simulink library "TripedLibraryMAX"  (R2021a)
settings: Solver=VariableStepAuto  StartTime=0.0  StopTime=10.0  RelTol=1e-3
undefined symbols (not defined in the file or in its folder): X, Y, Z
libraries: sm_lib x100, nesl_utility x31, $bdroot x27, robotlib x27, simulink x6
(part 3 of 6)

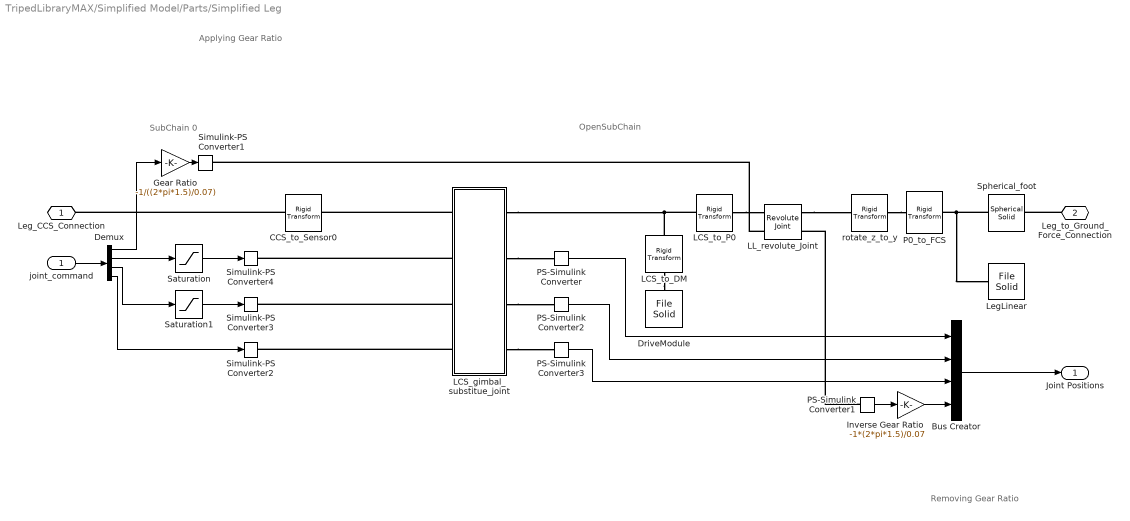
== system TripedLibraryMAX/Simplified Model/Parts/Simplified Leg ==
  note: Removing Gear Ratio
  note: Applying Gear Ratio
  note: SubChain 0
  note: OpenSubChain
  joint_command : Inport
  Bus Creator : BusCreator  Inputs=4
  CCS_to_Sensor0 : sm_lib/Frames and Transforms/Rigid Transform  TranslationMethod=Cartesian  TranslationStandardOffset=0  TranslationLengthUnit=mm  TranslationStandardAxis=+Z  TranslationCartesianOffset=[70 0 53]+[195 0 -39]  TranslationROffset=0  TranslationROffsetUnits=m  TranslationZOffset=0  TranslationZOffsetUnits=m  TranslationThetaOffset=0  TranslationThetaOffsetUnits=deg  RotationMethod=None  RotationAngleUnits=deg  RotationStandardAxis=+Z  RotationAngle=0.0  RotationArbitraryAxis=[0 0 1]  FollAlignAxisA=+X  BaseAlignAxisA=+Y  FollAlignAxisB=+Y  BaseAlignAxisB=+Z  RotationSequenceAxes=FollowerAxes  RotationSequence=XYX  RotationSequenceAngles=[0 0 0]  RotationSequenceAnglesUnits=deg  RotationMatrix=[1 0 0; 0 1 0; 0 0 1]
  Demux : Demux
  DriveModule : sm_lib/Body Elements/File Solid  ExtGeomFileName=./triped-files/TriPed_Bein_Antriebsmodul.stl  UnitType=Custom  ExtGeomFileUnits=mm  StepReaderType=HEX  ExportConvexHullGeometry=off  InertiaType=Custom  Mass=1.908  MassUnits=kg  CenterOfMass=[25.9 50.7 45.5]  CenterOfMassUnits=mm  MomentsOfInertia=[0.005 0.007 0.008]  MomentsOfInertiaUnits=kg*m^2  ProductsOfInertia=[0 0 0]  ProductsOfInertiaUnits=kg*m^2  BasedOnType=DensityFromFile  Density=1000  DensityUnits=kg/m^3  DoExposeReferenceFrame=on  SerializedFrames=<Frames/>  GraphicType=FromGeometry  MarkerShape=Sphere  MarkerSize=10  GraphicVisPropType=SimpleVisualProperties  GraphicDiffuseColor=[0.0 0.39607844 0.44705883]  GraphicSpecularColor=[0.5 0.5 0.5 1.0]  GraphicAmbientColor=[0.15 0.15 0.15 1.0]  GraphicEmissiveColor=[0.0 0.0 0.0 1.0]  GraphicShininess=75  GraphicOpacity=0.75
  Gear Ratio : Gain  Gain=-1/((2*pi*1.5)/0.07)
  Inverse Gear Ratio : Gain  Gain=-1*(2*pi*1.5)/0.07
  LCS_gimbal_substitue_joint : Subsystem
  LCS_to_DM : sm_lib/Frames and Transforms/Rigid Transform  TranslationMethod=Cartesian  TranslationStandardOffset=0  TranslationLengthUnit=mm  TranslationStandardAxis=+Z  TranslationCartesianOffset=[25.9 145 37]  TranslationROffset=0  TranslationROffsetUnits=m  TranslationZOffset=0  TranslationZOffsetUnits=m  TranslationThetaOffset=0  TranslationThetaOffsetUnits=deg  RotationMethod=StandardAxis  RotationAngleUnits=deg  RotationStandardAxis=+Z  RotationAngle=-90  RotationArbitraryAxis=[0 0 1]  FollAlignAxisA=+X  BaseAlignAxisA=+Y  FollAlignAxisB=+Y  BaseAlignAxisB=+Z  RotationSequenceAxes=FollowerAxes  RotationSequence=XYX  RotationSequenceAngles=[0 0 0]  RotationSequenceAnglesUnits=deg  RotationMatrix=[1 0 0; 0 1 0; 0 0 1]
  LCS_to_P0 : sm_lib/Frames and Transforms/Rigid Transform  TranslationMethod=Cartesian  TranslationStandardOffset=0  TranslationLengthUnit=mm  TranslationStandardAxis=+Z  TranslationCartesianOffset=[1640 0 -37]  TranslationROffset=0  TranslationROffsetUnits=m  TranslationZOffset=0  TranslationZOffsetUnits=m  TranslationThetaOffset=0  TranslationThetaOffsetUnits=deg  RotationMethod=StandardAxis  RotationAngleUnits=deg  RotationStandardAxis=+X  RotationAngle=-90  RotationArbitraryAxis=[0 0 1]  FollAlignAxisA=+X  BaseAlignAxisA=+Y  FollAlignAxisB=+Y  BaseAlignAxisB=+Z  RotationSequenceAxes=FollowerAxes  RotationSequence=XYX  RotationSequenceAngles=[0 0 0]  RotationSequenceAnglesUnits=deg  RotationMatrix=[1 0 0; 0 1 0; 0 0 1]
  LL_revolute_Joint : sm_lib/Joints/Revolute Joint  JointMode=Normal  SenseConstraintForce=off  SenseConstraintForceX=off  SenseConstraintForceY=off  SenseConstraintForceZ=off  SenseConstraintTorque=off  SenseConstraintTorqueX=off  SenseConstraintTorqueY=off  SenseConstraintTorqueZ=off  SenseTotalForce=off  SenseTotalForceX=off  SenseTotalForceY=off  SenseTotalForceZ=off  SenseTotalTorque=off  SenseTotalTorqueX=off  SenseTotalTorqueY=off  SenseTotalTorqueZ=off  CompositeWrenchDir=FollowerOnBase  CompositeWrenchFrame=BaseFrame  EquilibriumPosition=0  EquilibriumPositionUnits=deg  SpringStiffness=0  SpringStiffnessUnits=N*m/deg  DampingCoefficient=0  DampingCoefficientUnits=N*m/(deg/s)  TorqueActuationMode=ComputedTorque  MotionActuationMode=InputMotion  SensePosition=on  SenseVelocity=off  SenseAcceleration=off  SenseTorqueForce=off  SenseLowerLimitTorqueForce=off  SenseUpperLimitTorqueForce=off  LowerLimitSpecify=off  LowerLimitBound=-60  LowerLimitBoundUnits=deg  LowerLimitStiffness=1e4  LowerLimitStiffnessUnits=N*m/deg  LowerLimitDamping=10  LowerLimitDampingUnits=N*m/(deg/s)  LowerLimitTransitionRegionWidth=0.1  LowerLimitTransitionRegionWidthUnits=deg  UpperLimitSpecify=off  UpperLimitBound=30  UpperLimitBoundUnits=deg  UpperLimitStiffness=1e4  UpperLimitStiffnessUnits=N*m/deg  UpperLimitDamping=1e4  UpperLimitDampingUnits=N*m/(deg/s)  UpperLimitTransitionRegionWidth=0.1  UpperLimitTransitionRegionWidthUnits=deg  PositionTargetSpecify=off  PositionTargetPriority=High  PositionTargetValue=0  PositionTargetValueUnits=deg  VelocityTargetSpecify=off  VelocityTargetPriority=High  VelocityTargetValue=0  VelocityTargetValueUnits=deg/s
  LegLinear : sm_lib/Body Elements/File Solid  ExtGeomFileName=./triped-files/TriPed_Bein_Linearteil_überarbeitet.stl  UnitType=Custom  ExtGeomFileUnits=mm  StepReaderType=HEX  ExportConvexHullGeometry=on  InertiaType=Custom  Mass=0.503  MassUnits=kg  CenterOfMass=[94.275 0 452.725]  CenterOfMassUnits=mm  MomentsOfInertia=[0.0006157 0.047 0.047]  MomentsOfInertiaUnits=kg*m^2  ProductsOfInertia=[0 0 0]  ProductsOfInertiaUnits=kg*m^2  BasedOnType=DensityFromFile  Density=1000  DensityUnits=kg/m^3  DoExposeReferenceFrame=on  SerializedFrames=<Frames/>  GraphicType=FromGeometry  MarkerShape=Sphere  MarkerSize=10  GraphicVisPropType=SimpleVisualProperties  GraphicDiffuseColor=[0.60784316 0.6509804 0.05882353]  GraphicSpecularColor=[0.5 0.5 0.5 1.0]  GraphicAmbientColor=[0.15 0.15 0.15 1.0]  GraphicEmissiveColor=[0.0 0.0 0.0 1.0]  GraphicShininess=75  GraphicOpacity=1.0
  P0_to_FCS : sm_lib/Frames and Transforms/Rigid Transform  TranslationMethod=StandardAxis  TranslationStandardOffset=-1500  TranslationLengthUnit=mm  TranslationStandardAxis=+X  TranslationCartesianOffset=[0 0 0]  TranslationROffset=0  TranslationROffsetUnits=m  TranslationZOffset=0  TranslationZOffsetUnits=m  TranslationThetaOffset=0  TranslationThetaOffsetUnits=deg  RotationMethod=None  RotationAngleUnits=deg  RotationStandardAxis=+Z  RotationAngle=0.0  RotationArbitraryAxis=[0 0 1]  FollAlignAxisA=+X  BaseAlignAxisA=+Y  FollAlignAxisB=+Y  BaseAlignAxisB=+Z  RotationSequenceAxes=FollowerAxes  RotationSequence=XYX  RotationSequenceAngles=[0 0 0]  RotationSequenceAnglesUnits=deg  RotationMatrix=[1 0 0; 0 1 0; 0 0 1]
  PS-Simulink Converter{,1..3} (4 alike) : nesl_utility/PS-Simulink Converter  PhysicalDomain=network_engine_domain  SubClassName=ps_output  LeftPortType=input  RightPortType=output  PseudoPeriodic=off  Frequencies=[]  Unit=inherit  AffineConversion=off
      settings: SourceProductBaseCode=PW,SS
  Saturation : Saturate  UpperLimit=0.523599  LowerLimit=-0.523599
  Saturation1 : Saturate  UpperLimit=0.523599  LowerLimit=-0.523599
  Simulink-PS Converter{1..4} (4 alike) : nesl_utility/Simulink-PS Converter  PhysicalDomain=network_engine_domain  SubClassName=ps_input  LeftPortType=input  RightPortType=output  PseudoPeriodic=off  Frequencies=[]  NoiseDistribution=none  NoiseParameters=[]  Unit=1  AffineConversion=off  FilteringAndDerivatives=filter  SimscapeFilterOrder=2  InputFilterTimeConstant=0.01  UdotUserProvided=0
      settings: SourceProductBaseCode=PW,SS
  Spherical_foot : sm_lib/Body Elements/Spherical Solid  GraphicAmbientColor=[0.15 0.15 0.15 1.0]  BasedOnType=Density  CenterOfMass=[0 0 0]  CenterOfMassUnits=m  GraphicDiffuseColor=[1.0 0.63529414 0.0]  Density=0  DensityUnits=kg/m^3  DoExposeReferenceFrame=on  GraphicEmissiveColor=[0.0 0.0 0.0 1.0]  ExportEntireGeometry=on  SerializedExposedGeometries=<ExposedGeometries><ExposedGeometry><RawGeometryId>entire</RawGeometryId><ExposedGeometryId>G</ExposedGeometryId></ExposedGeometry></ExposedGeometries>  SerializedFrames=<Frames/>  MarkerShape=Sphere  MarkerSize=10  GraphicType=FromGeometry  InertiaType=CalculateFromGeometry  Mass=1  MassUnits=kg  MomentsOfInertia=[1 1 1]  MomentsOfInertiaUnits=kg*m^2  GraphicOpacity=1.0  ProductsOfInertia=[0 0 0]  ProductsOfInertiaUnits=kg*m^2  GraphicShininess=75  GraphicSpecularColor=[0.5 0.5 0.5 1.0]  SphereRadius=0.03  SphereRadiusUnits=m  GraphicVisPropType=SimpleVisualProperties
  rotate_z_to_y : sm_lib/Frames and Transforms/Rigid Transform  TranslationMethod=None  TranslationStandardOffset=0  TranslationLengthUnit=m  TranslationStandardAxis=+Z  TranslationCartesianOffset=[0 0 0]  TranslationROffset=0  TranslationROffsetUnits=m  TranslationZOffset=0  TranslationZOffsetUnits=m  TranslationThetaOffset=0  TranslationThetaOffsetUnits=deg  RotationMethod=StandardAxis  RotationAngleUnits=deg  RotationStandardAxis=+X  RotationAngle=90  RotationArbitraryAxis=[0 0 1]  FollAlignAxisA=+X  BaseAlignAxisA=+Y  FollAlignAxisB=+Y  BaseAlignAxisB=+Z  RotationSequenceAxes=FollowerAxes  RotationSequence=XYX  RotationSequenceAngles=[0 0 0]  RotationSequenceAnglesUnits=deg  RotationMatrix=[1 0 0; 0 1 0; 0 0 1]
  Leg_CCS_Connection : PMIOPort  Side=Left
  Leg_to_Ground_Force_Connection : PMIOPort  Port=2  Side=Left
  Joint Positions : Outport
  wires:
  PS-Simulink Converter3/1 -> Bus Creator/3
  PS-Simulink Converter2/1 -> Bus Creator/2
  Inverse Gear Ratio/1 -> Bus Creator/4
  Demux/4 -> Simulink-PS Converter2/1
  Demux/3 -> Saturation1/1
  PS-Simulink Converter/1 -> Bus Creator/1
  Gear Ratio/1 -> Simulink-PS Converter1/1
  Bus Creator/1 -> Joint Positions/1
  PS-Simulink Converter1/1 -> Inverse Gear Ratio/1
  joint_command/1 -> Demux/1
  Demux/1 -> Gear Ratio/1
  Demux/2 -> Saturation/1
  Saturation/1 -> Simulink-PS Converter4/1
  Saturation1/1 -> Simulink-PS Converter3/1
  connections:
  P0_to_FCS/RConn1 -- Spherical_foot/RConn1 -- LegLinear/RConn1
  LCS_to_P0/LConn1 -- LCS_gimbal_substitue_joint/RConn1 -- LCS_to_DM/RConn1
  rotate_z_to_y/RConn1 -- P0_to_FCS/LConn1
  LCS_gimbal_substitue_joint/LConn4 -- Simulink-PS Converter2/RConn1
  LCS_gimbal_substitue_joint/RConn3 -- PS-Simulink Converter2/LConn1
  LCS_gimbal_substitue_joint/LConn2 -- Simulink-PS Converter4/RConn1
  LL_revolute_Joint/LConn2 -- Simulink-PS Converter1/RConn1
  LCS_gimbal_substitue_joint/LConn3 -- Simulink-PS Converter3/RConn1
  LCS_to_P0/RConn1 -- LL_revolute_Joint/LConn1
  LCS_gimbal_substitue_joint/RConn2 -- PS-Simulink Converter/LConn1
  LCS_gimbal_substitue_joint/RConn4 -- PS-Simulink Converter3/LConn1
  LCS_to_DM/LConn1 -- DriveModule/RConn1
  Leg_to_Ground_Force_Connection/RConn1 -- Spherical_foot/LConn1
  LL_revolute_Joint/RConn1 -- rotate_z_to_y/LConn1
  LCS_gimbal_substitue_joint/LConn1 -- CCS_to_Sensor0/RConn1
  PS-Simulink Converter1/LConn1 -- LL_revolute_Joint/RConn2
  CCS_to_Sensor0/LConn1 -- Leg_CCS_Connection/RConn1

== system TripedLibraryMAX/Simplified Model/Simplified TriPed/Chassis ==
  Chassis : sm_lib/Body Elements/File Solid  ExtGeomFileName=./triped-files/TriPed_Chassis_überarbeitet.stl  UnitType=Custom  ExtGeomFileUnits=mm  StepReaderType=HEX  ExportConvexHullGeometry=off  InertiaType=Custom  Mass=10.414  MassUnits=kg  CenterOfMass=[0 0 10.44]  CenterOfMassUnits=mm  MomentsOfInertia=[0.127 0.127 0.209]  MomentsOfInertiaUnits=kg*m^2  ProductsOfInertia=[0 0 0]  ProductsOfInertiaUnits=kg*m^2  BasedOnType=DensityFromFile  Density=1000  DensityUnits=kg/m^3  DoExposeReferenceFrame=on  SerializedFrames=<Frames/>  GraphicType=FromGeometry  MarkerShape=Sphere  MarkerSize=10  GraphicVisPropType=SimpleVisualProperties  GraphicDiffuseColor=[1.0 0.63529414 0.0]  GraphicSpecularColor=[0.5 0.5 0.5 1.0]  GraphicAmbientColor=[0.15 0.15 0.15 1.0]  GraphicEmissiveColor=[0.0 0.0 0.0 1.0]  GraphicShininess=75  GraphicOpacity=1
  visualize CCS : Subsystem
  " Chassis_connection" : PMIOPort  Side=Left
  Forward Kin Visual : PMIOPort  Port=2  Side=Right
  connections:
  Chassis/RConn1 -- visualize CCS/LConn1 -- Forward Kin Visual/RConn1 -- " Chassis_connection"/RConn1

== system TripedLibraryMAX/Simplified Model/Simplified TriPed (Kinematics)/Chassis ==
  Chassis : sm_lib/Body Elements/File Solid  ExtGeomFileName=./triped-files/TriPed_Chassis_überarbeitet.stl  UnitType=Custom  ExtGeomFileUnits=mm  StepReaderType=HEX  ExportConvexHullGeometry=off  InertiaType=Custom  Mass=10.414  MassUnits=kg  CenterOfMass=[0 0 10.44]  CenterOfMassUnits=mm  MomentsOfInertia=[0.127 0.127 0.209]  MomentsOfInertiaUnits=kg*m^2  ProductsOfInertia=[0 0 0]  ProductsOfInertiaUnits=kg*m^2  BasedOnType=DensityFromFile  Density=1000  DensityUnits=kg/m^3  DoExposeReferenceFrame=on  SerializedFrames=<Frames/>  GraphicType=FromGeometry  MarkerShape=Sphere  MarkerSize=10  GraphicVisPropType=SimpleVisualProperties  GraphicDiffuseColor=[1.0 0.63529414 0.0]  GraphicSpecularColor=[0.5 0.5 0.5 1.0]  GraphicAmbientColor=[0.15 0.15 0.15 1.0]  GraphicEmissiveColor=[0.0 0.0 0.0 1.0]  GraphicShininess=75  GraphicOpacity=1
  visualize CCS : Subsystem
  " Chassis_connection" : PMIOPort  Side=Left
  Forward Kin Visual : PMIOPort  Port=2  Side=Right
  connections:
  Chassis/RConn1 -- visualize CCS/LConn1 -- Forward Kin Visual/RConn1 -- " Chassis_connection"/RConn1

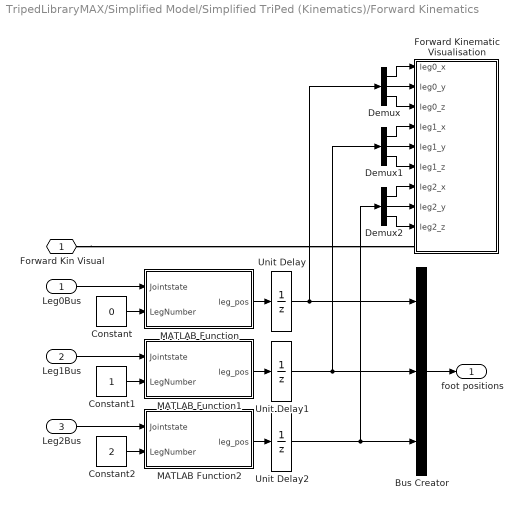
== system TripedLibraryMAX/Simplified Model/Simplified TriPed (Kinematics)/Forward Kinematics ==
  Leg0Bus : Inport
  Leg1Bus : Inport  Port=2
  Leg2Bus : Inport  Port=3
  Bus Creator : BusCreator  Inputs=3
  Constant : Constant  Value=0
  Constant1 : Constant
  Constant2 : Constant  Value=2
  Demux : Demux  Outputs=3
  Demux1 : Demux  Outputs=3
  Demux2 : Demux  Outputs=3
  Forward Kinematic Visualisation : Subsystem
  MATLAB Function : MATLAB Function  TreatAsAtomicUnit=on
  MATLAB Function1 : MATLAB Function  TreatAsAtomicUnit=on
  MATLAB Function2 : MATLAB Function  TreatAsAtomicUnit=on
  Unit Delay : UnitDelay  InitialCondition=[0 0 0]  SampleTime=-1  HasFrameUpgradeWarning=on
  Unit Delay1 : UnitDelay  InitialCondition=[0 0 0]  SampleTime=-1  HasFrameUpgradeWarning=on
  Unit Delay2 : UnitDelay  InitialCondition=[0 0 0]  SampleTime=-1  HasFrameUpgradeWarning=on
  Forward Kin Visual : PMIOPort  Side=Right
  foot positions : Outport
  wires:
  Leg0Bus/1 -> MATLAB Function/1(Jointstate)
  Leg1Bus/1 -> MATLAB Function1/1(Jointstate)
  Leg2Bus/1 -> MATLAB Function2/1(Jointstate)
  Bus Creator/1 -> foot positions/1
  Constant/1 -> MATLAB Function/2(LegNumber)
  Constant1/1 -> MATLAB Function1/2(LegNumber)
  Constant2/1 -> MATLAB Function2/2(LegNumber)
  Unit Delay2/1 -> Demux2/1, Bus Creator/3
  MATLAB Function2/1(leg_pos) -> Unit Delay2/1
  MATLAB Function1/1(leg_pos) -> Unit Delay1/1
  Unit Delay1/1 -> Demux1/1, Bus Creator/2
  Unit Delay/1 -> Demux/1, Bus Creator/1
  MATLAB Function/1(leg_pos) -> Unit Delay/1
  Demux/1 -> Forward Kinematic Visualisation/1(leg0_x)
  Demux/2 -> Forward Kinematic Visualisation/2(leg0_y)
  Demux/3 -> Forward Kinematic Visualisation/3(leg0_z)
  Demux1/2 -> Forward Kinematic Visualisation/5(leg1_y)
  Demux1/1 -> Forward Kinematic Visualisation/4(leg1_x)
  Demux1/3 -> Forward Kinematic Visualisation/6(leg1_z)
  Demux2/1 -> Forward Kinematic Visualisation/7(leg2_x)
  Demux2/2 -> Forward Kinematic Visualisation/8(leg2_y)
  Demux2/3 -> Forward Kinematic Visualisation/9(leg2_z)
  connections:
  Forward Kin Visual/RConn1 -- Forward Kinematic Visualisation/LConn1

== system TripedLibraryMAX/Simplified Model/Simplified TriPed (Kinematics)/Inverse Kinematics ==
  note: Inverse Kinematics Target Visualisation
  note: Inverse Kinematics
  In1 : Inport
  Constant : Constant  Value=0
  Constant1 : Constant  Value=120*pi/180
  Constant2 : Constant  Value=240*pi/180
  Demux : Demux  Outputs=9
  Inv Kin : $bdroot/Kinematic Utils/Simplified  Model Kinematics/Inv Kin  SystemSampleTime=-1  VariantActivationTime=update diagram  Latency=0  AutoFrameSizeCalculation=off
  Inv Kin1 : $bdroot/Kinematic Utils/Simplified  Model Kinematics/Inv Kin  SystemSampleTime=-1  VariantActivationTime=update diagram  Latency=0  AutoFrameSizeCalculation=off
  Inv Kin2 : $bdroot/Kinematic Utils/Simplified  Model Kinematics/Inv Kin  SystemSampleTime=-1  VariantActivationTime=update diagram  Latency=0  AutoFrameSizeCalculation=off
  Inverse Kinematic Visualisation : Subsystem
  vis. : PMIOPort  Side=Left
  Leg0Bus : Outport
  Leg1Bus : Outport  Port=2
  Leg2Bus : Outport  Port=3
  wires:
  Demux/7 -> Inv Kin2/1, Inverse Kinematic Visualisation/7(leg2_x)
  Constant1/1 -> Inv Kin1/4
  Demux/9 -> Inv Kin2/3, Inverse Kinematic Visualisation/9(leg2_z)
  Constant/1 -> Inv Kin/4
  Constant2/1 -> Inv Kin2/4
  Demux/5 -> Inv Kin1/2, Inverse Kinematic Visualisation/5(leg1_y)
  Inv Kin2/1 -> Leg2Bus/1
  Demux/4 -> Inv Kin1/1, Inverse Kinematic Visualisation/4(leg1_x)
  Demux/6 -> Inv Kin1/3, Inverse Kinematic Visualisation/6(leg1_z)
  Demux/3 -> Inv Kin/3, Inverse Kinematic Visualisation/3(leg0_z)
  Demux/8 -> Inv Kin2/2, Inverse Kinematic Visualisation/8(leg2_y)
  Demux/2 -> Inv Kin/2, Inverse Kinematic Visualisation/2(leg0_y)
  Inv Kin/1 -> Leg0Bus/1
  Inv Kin1/1 -> Leg1Bus/1
  In1/1 -> Demux/1
  Demux/1 -> Inv Kin/1, Inverse Kinematic Visualisation/1(leg0_x)
  connections:
  vis./RConn1 -- Inverse Kinematic Visualisation/LConn1

== system TripedLibraryMAX/Full Model/Legs and Body/Leg/Sensor Modelling ==
  Extend Sensor : $bdroot/Full Model/Sensors/Extend Sensor  SystemSampleTime=-1  VariantActivationTime=update diagram  Latency=0  AutoFrameSizeCalculation=off
  PS-Simulink Converter : nesl_utility/PS-Simulink Converter  PhysicalDomain=network_engine_domain  SubClassName=ps_output  LeftPortType=input  RightPortType=output  PseudoPeriodic=off  Frequencies=[]  Unit=inherit  AffineConversion=off
      settings: SourceProductBaseCode=PW,SS
  PS-Simulink Converter1 : nesl_utility/PS-Simulink Converter  PhysicalDomain=network_engine_domain  SubClassName=ps_output  LeftPortType=input  RightPortType=output  PseudoPeriodic=off  Frequencies=[]  Unit=inherit  AffineConversion=off
      settings: SourceProductBaseCode=PW,SS
  PS-Simulink Converter2 : nesl_utility/PS-Simulink Converter  PhysicalDomain=network_engine_domain  SubClassName=ps_output  LeftPortType=input  RightPortType=output  PseudoPeriodic=off  Frequencies=[]  Unit=inherit  AffineConversion=off
      settings: SourceProductBaseCode=PW,SS
  Swing Left Sensor : $bdroot/Full Model/Sensors/Swing Sensor  SystemSampleTime=-1  VariantActivationTime=update diagram  Latency=0  AutoFrameSizeCalculation=off
  Swing Right Sensor : $bdroot/Full Model/Sensors/Swing Sensor  SystemSampleTime=-1  VariantActivationTime=update diagram  Latency=0  AutoFrameSizeCalculation=off
  negate Gear conversion : Gain  Gain=1/(2*pi*1.5)*0.07
  swing left : PMIOPort  Side=Left
  extend motor : PMIOPort  Port=2  Side=Left
  swing right : PMIOPort  Port=3  Side=Left
  sls_quant : Outport
  ses_quant : Outport  Port=2
  srs_quant : Outport  Port=3
  wires:
  PS-Simulink Converter/1 -> Swing Left Sensor/1
  Swing Left Sensor/1 -> sls_quant/1
  PS-Simulink Converter2/1 -> Extend Sensor/1
  Extend Sensor/1 -> ses_quant/1
  PS-Simulink Converter1/1 -> Swing Right Sensor/1
  Swing Right Sensor/1 -> srs_quant/1
  connections:
  swing left/RConn1 -- PS-Simulink Converter/LConn1
  swing right/RConn1 -- PS-Simulink Converter1/LConn1
  extend motor/RConn1 -- PS-Simulink Converter2/LConn1

== system TripedLibraryMAX/Full Model/Legs and Body/Leg/motor-joint conversion ==
  joint commands : Inport
  Demux : Demux  Outputs=3
  extend  conversion : Gain  Gain=-(2*pi*1.5)/0.07
  swing left conversion : Gain  Gain=50
  swing right conversion : Gain  Gain=50
  swing/left : Outport
  extend : Outport  Port=2
  swing/right : Outport  Port=3
  wires:
  swing left conversion/1 -> swing/left/1
  swing right conversion/1 -> swing/right/1
  Demux/3 -> swing right conversion/1
  joint commands/1 -> Demux/1
  extend  conversion/1 -> extend/1
  Demux/2 -> extend  conversion/1
  Demux/1 -> swing left conversion/1

== system TripedLibraryMAX/Full Model/ROS/Publishers/Publisher_leg_0 ==
  Leg_0_jointstate : Inport
  Demux : Demux  Outputs=3
  leg_0_extend_joint_pub : Subsystem  mask(Motor Publisher){topic=/leg_0/extend/joint/joint_states}
      mask prompts: topic "ros:slros:blockmask:TopicPrompt"
      mask help: This is a sample description
      mask TypeOptions topic: RosPublisher/topic
      mask DialogControl topic Prompt: Topic:
      mask Display: block_icon('RosPublisher')
  leg_0_swing_left_joint_pub : Subsystem  mask(Motor Publisher){topic=/leg_0/swing/left/joint/joint_states}
      mask prompts: topic "ros:slros:blockmask:TopicPrompt"
      mask help: This is a sample description
      mask TypeOptions topic: RosPublisher/topic
      mask DialogControl topic Prompt: Topic:
      mask Display: block_icon('RosPublisher')
  leg_0_swing_right_joint_pub : Subsystem  mask(Motor Publisher){topic=/leg_0/swing/right/joint/joint_states}
      mask prompts: topic "ros:slros:blockmask:TopicPrompt"
      mask help: This is a sample description
      mask TypeOptions topic: RosPublisher/topic
      mask DialogControl topic Prompt: Topic:
      mask Display: block_icon('RosPublisher')
  wires:
  Leg_0_jointstate/1 -> Demux/1
  Demux/3 -> leg_0_swing_right_joint_pub/1(position)
  Demux/2 -> leg_0_extend_joint_pub/1(position)
  Demux/1 -> leg_0_swing_left_joint_pub/1(position)

== system TripedLibraryMAX/Full Model/ROS/Publishers/Publisher_leg_1 ==
  Leg_1_jointstate : Inport
  Demux : Demux  Outputs=3
  leg_1_extend_joint_pub : Subsystem  mask(Motor Publisher){topic=/leg_1/extend/joint/joint_states}
      mask prompts: topic "ros:slros:blockmask:TopicPrompt"
      mask help: This is a sample description
      mask TypeOptions topic: RosPublisher/topic
      mask DialogControl topic Prompt: Topic:
      mask Display: block_icon('RosPublisher')
  leg_1_swing_left_joint_pub : Subsystem  mask(Motor Publisher){topic=/leg_1/swing/left/joint/joint_states}
      mask prompts: topic "ros:slros:blockmask:TopicPrompt"
      mask help: This is a sample description
      mask TypeOptions topic: RosPublisher/topic
      mask DialogControl topic Prompt: Topic:
      mask Display: block_icon('RosPublisher')
  leg_1_swing_right_joint_pub : Subsystem  mask(Motor Publisher){topic=/leg_1/swing/right/joint/joint_states}
      mask prompts: topic "ros:slros:blockmask:TopicPrompt"
      mask help: This is a sample description
      mask TypeOptions topic: RosPublisher/topic
      mask DialogControl topic Prompt: Topic:
      mask Display: block_icon('RosPublisher')
  wires:
  Leg_1_jointstate/1 -> Demux/1
  Demux/3 -> leg_1_swing_right_joint_pub/1(position)
  Demux/2 -> leg_1_extend_joint_pub/1(position)
  Demux/1 -> leg_1_swing_left_joint_pub/1(position)

== system TripedLibraryMAX/Full Model/ROS/Publishers/Publisher_leg_2 ==
  Leg_2_jointstate : Inport
  Demux : Demux  Outputs=3
  leg_2_extend_joint_pub : Subsystem  mask(Motor Publisher){topic=/leg_2/extend/joint/joint_states}
      mask prompts: topic "ros:slros:blockmask:TopicPrompt"
      mask help: This is a sample description
      mask TypeOptions topic: RosPublisher/topic
      mask DialogControl topic Prompt: Topic:
      mask Display: block_icon('RosPublisher')
  leg_2_swing_left_joint_pub : Subsystem  mask(Motor Publisher){topic=/leg_2/swing/left/joint/joint_states}
      mask prompts: topic "ros:slros:blockmask:TopicPrompt"
      mask help: This is a sample description
      mask TypeOptions topic: RosPublisher/topic
      mask DialogControl topic Prompt: Topic:
      mask Display: block_icon('RosPublisher')
  leg_2_swing_right_joint_pub : Subsystem  mask(Motor Publisher){topic=/leg_2/swing/right/joint/joint_states}
      mask prompts: topic "ros:slros:blockmask:TopicPrompt"
      mask help: This is a sample description
      mask TypeOptions topic: RosPublisher/topic
      mask DialogControl topic Prompt: Topic:
      mask Display: block_icon('RosPublisher')
  wires:
  Leg_2_jointstate/1 -> Demux/1
  Demux/3 -> leg_2_swing_right_joint_pub/1(position)
  Demux/2 -> leg_2_extend_joint_pub/1(position)
  Demux/1 -> leg_2_swing_left_joint_pub/1(position)

== system TripedLibraryMAX/Full Model/ROS/Subscribers/Subscriber_leg0 ==
  Mux : Mux  Inputs=3
  Ros-Subscriber-leg-0-extend-motor : Subsystem
  Ros-Subscriber-leg-0-left-swing-motor : Subsystem
  Ros-Subscriber-leg-0-right-swing-motor : Subsystem
  Leg_0_joint_commands : Outport
  wires:
  Ros-Subscriber-leg-0-left-swing-motor/1(swing_left_motor_position_OUT) -> Mux/1
  Mux/1 -> Leg_0_joint_commands/1
  Ros-Subscriber-leg-0-extend-motor/1(extend_motor_position_OUT) -> Mux/2
  Ros-Subscriber-leg-0-right-swing-motor/1(swing_right_motor_position_OUT) -> Mux/3

== system TripedLibraryMAX/Full Model/ROS/Subscribers/Subscriber_leg1 ==
  Mux : Mux  Inputs=3
  Ros-Subscriber-leg-1-extend-motor : Subsystem
  Ros-Subscriber-leg-1-left-swing-motor : Subsystem
  Ros-Subscriber-leg-1-right-swing-motor : Subsystem
  Leg_1_joint_commands : Outport
  wires:
  Ros-Subscriber-leg-1-left-swing-motor/1(swing_left_motor_position_OUT) -> Mux/1
  Mux/1 -> Leg_1_joint_commands/1
  Ros-Subscriber-leg-1-extend-motor/1(extend_motor_position_OUT) -> Mux/2
  Ros-Subscriber-leg-1-right-swing-motor/1(swing_right_motor_position_OUT) -> Mux/3

== system TripedLibraryMAX/Full Model/ROS/Subscribers/Subscriber_leg2 ==
  Mux : Mux  Inputs=3
  Ros-Subscriber-leg-2-extend-motor : Subsystem
  Ros-Subscriber-leg-2-left-swing-motor : Subsystem
  Ros-Subscriber-leg-2-right-swing-motor : Subsystem
  Leg_2_joint_commands : Outport
  wires:
  Ros-Subscriber-leg-2-left-swing-motor/1(swing_left_motor_position_OUT) -> Mux/1
  Mux/1 -> Leg_2_joint_commands/1
  Ros-Subscriber-leg-2-extend-motor/1(extend_motor_position_OUT) -> Mux/2
  Ros-Subscriber-leg-2-right-swing-motor/1(swing_right_motor_position_OUT) -> Mux/3

== system TripedLibraryMAX/Full Model/TriPed/Chassis/trafo chassis to center ==
  Rigid Transform : sm_lib/Frames and Transforms/Rigid Transform  TranslationMethod=None  TranslationStandardOffset=0  TranslationLengthUnit=m  TranslationStandardAxis=+Z  TranslationCartesianOffset=[0 0 0]  TranslationROffset=0  TranslationROffsetUnits=m  TranslationZOffset=0  TranslationZOffsetUnits=m  TranslationThetaOffset=0  TranslationThetaOffsetUnits=deg  RotationMethod=StandardAxis  RotationAngleUnits=deg  RotationStandardAxis=-X  RotationAngle=90  RotationArbitraryAxis=[0 0 1]  FollAlignAxisA=+X  BaseAlignAxisA=+Y  FollAlignAxisB=+Y  BaseAlignAxisB=+Z  RotationSequenceAxes=FollowerAxes  RotationSequence=XYX  RotationSequenceAngles=[0 0 0]  RotationSequenceAnglesUnits=deg  RotationMatrix=[1 0 0; 0 1 0; 0 0 1]
  Rigid Transform1 : sm_lib/Frames and Transforms/Rigid Transform  TranslationMethod=None  TranslationStandardOffset=0  TranslationLengthUnit=m  TranslationStandardAxis=+Z  TranslationCartesianOffset=[0 0 0]  TranslationROffset=0  TranslationROffsetUnits=m  TranslationZOffset=0  TranslationZOffsetUnits=m  TranslationThetaOffset=0  TranslationThetaOffsetUnits=deg  RotationMethod=StandardAxis  RotationAngleUnits=deg  RotationStandardAxis=-Y  RotationAngle=90  RotationArbitraryAxis=[0 0 1]  FollAlignAxisA=+X  BaseAlignAxisA=+Y  FollAlignAxisB=+Y  BaseAlignAxisB=+Z  RotationSequenceAxes=FollowerAxes  RotationSequence=XYX  RotationSequenceAngles=[0 0 0]  RotationSequenceAnglesUnits=deg  RotationMatrix=[1 0 0; 0 1 0; 0 0 1]
  Rigid Transform2 : sm_lib/Frames and Transforms/Rigid Transform  TranslationMethod=StandardAxis  TranslationStandardOffset=0.26  TranslationLengthUnit=m  TranslationStandardAxis=-Z  TranslationCartesianOffset=[0 0 0]  TranslationROffset=0  TranslationROffsetUnits=m  TranslationZOffset=0  TranslationZOffsetUnits=m  TranslationThetaOffset=0  TranslationThetaOffsetUnits=deg  RotationMethod=None  RotationAngleUnits=deg  RotationStandardAxis=-Y  RotationAngle=90  RotationArbitraryAxis=[0 0 1]  FollAlignAxisA=+X  BaseAlignAxisA=+Y  FollAlignAxisB=+Y  BaseAlignAxisB=+Z  RotationSequenceAxes=FollowerAxes  RotationSequence=XYX  RotationSequenceAngles=[0 0 0]  RotationSequenceAnglesUnits=deg  RotationMatrix=[1 0 0; 0 1 0; 0 0 1]
  Rigid Transform3 : sm_lib/Frames and Transforms/Rigid Transform  TranslationMethod=StandardAxis  TranslationStandardOffset=0.02  TranslationLengthUnit=m  TranslationStandardAxis=-Y  TranslationCartesianOffset=[0 0 0]  TranslationROffset=0  TranslationROffsetUnits=m  TranslationZOffset=0  TranslationZOffsetUnits=m  TranslationThetaOffset=0  TranslationThetaOffsetUnits=deg  RotationMethod=None  RotationAngleUnits=deg  RotationStandardAxis=-Y  RotationAngle=90  RotationArbitraryAxis=[0 0 1]  FollAlignAxisA=+X  BaseAlignAxisA=+Y  FollAlignAxisB=+Y  BaseAlignAxisB=+Z  RotationSequenceAxes=FollowerAxes  RotationSequence=XYX  RotationSequenceAngles=[0 0 0]  RotationSequenceAnglesUnits=deg  RotationMatrix=[1 0 0; 0 1 0; 0 0 1]
  Conn1 : PMIOPort  Side=Left
  Conn2 : PMIOPort  Port=2  Side=Right
  connections:
  Rigid Transform3/LConn1 -- Rigid Transform2/RConn1
  Rigid Transform/RConn1 -- Rigid Transform1/LConn1
  Rigid Transform3/RConn1 -- Conn2/RConn1
  Rigid Transform/LConn1 -- Conn1/RConn1
  Rigid Transform1/RConn1 -- Rigid Transform2/LConn1

== system TripedLibraryMAX/Full Model/TriPed/Chassis/visualize CCS ==
  Rotate_Leg : sm_lib/Frames and Transforms/Rigid Transform  TranslationMethod=None  TranslationStandardOffset=0  TranslationLengthUnit=m  TranslationStandardAxis=+Z  TranslationCartesianOffset=[0 0 0]  TranslationROffset=0  TranslationROffsetUnits=m  TranslationZOffset=0  TranslationZOffsetUnits=m  TranslationThetaOffset=0  TranslationThetaOffsetUnits=deg  RotationMethod=StandardAxis  RotationAngleUnits=deg  RotationStandardAxis=+X  RotationAngle=90  RotationArbitraryAxis=[0 0 1]  FollAlignAxisA=+X  BaseAlignAxisA=+Y  FollAlignAxisB=+Y  BaseAlignAxisB=+Z  RotationSequenceAxes=FollowerAxes  RotationSequence=XYX  RotationSequenceAngles=[0 0 0]  RotationSequenceAnglesUnits=deg  RotationMatrix=[1 0 0; 0 1 0; 0 0 1]
  Rotate_Leg1 : sm_lib/Frames and Transforms/Rigid Transform  TranslationMethod=None  TranslationStandardOffset=0  TranslationLengthUnit=m  TranslationStandardAxis=+Z  TranslationCartesianOffset=[0 0 0]  TranslationROffset=0  TranslationROffsetUnits=m  TranslationZOffset=0  TranslationZOffsetUnits=m  TranslationThetaOffset=0  TranslationThetaOffsetUnits=deg  RotationMethod=StandardAxis  RotationAngleUnits=deg  RotationStandardAxis=+Y  RotationAngle=90  RotationArbitraryAxis=[0 0 1]  FollAlignAxisA=+X  BaseAlignAxisA=+Y  FollAlignAxisB=+Y  BaseAlignAxisB=+Z  RotationSequenceAxes=FollowerAxes  RotationSequence=XYX  RotationSequenceAngles=[0 0 0]  RotationSequenceAnglesUnits=deg  RotationMatrix=[1 0 0; 0 1 0; 0 0 1]
  X Axis : sm_lib/Body Elements/Cylindrical Solid  GraphicAmbientColor=[0.15 0.15 0.15 1.0]  BasedOnType=Density  CenterOfMass=[0 0 0]  CenterOfMassUnits=m  GraphicDiffuseColor=[1.0 0.0 0.0]  CylinderLength=0.8  CylinderLengthUnits=m  CylinderRadius=0.01  CylinderRadiusUnits=m  Density=1  DensityUnits=kg/m^3  DoExposeReferenceFrame=on  GraphicEmissiveColor=[0.0 0.0 0.0 1.0]  ExportEntireGeometry=off  SerializedFrames=<Frames/>  MarkerShape=Sphere  MarkerSize=10  GraphicType=FromGeometry  InertiaType=CalculateFromGeometry  Mass=1  MassUnits=kg  MomentsOfInertia=[1 1 1]  MomentsOfInertiaUnits=kg*m^2  GraphicOpacity=1.0  ProductsOfInertia=[0 0 0]  ProductsOfInertiaUnits=kg*m^2  GraphicShininess=75  GraphicSpecularColor=[0.5 0.5 0.5 1.0]  GraphicVisPropType=SimpleVisualProperties
  Y axis : sm_lib/Body Elements/Cylindrical Solid  GraphicAmbientColor=[0.15 0.15 0.15 1.0]  BasedOnType=Density  CenterOfMass=[0 0 0]  CenterOfMassUnits=m  GraphicDiffuseColor=[0.0 1.0 0.0]  CylinderLength=0.8  CylinderLengthUnits=m  CylinderRadius=0.01  CylinderRadiusUnits=m  Density=1  DensityUnits=kg/m^3  DoExposeReferenceFrame=on  GraphicEmissiveColor=[0.0 0.0 0.0 1.0]  ExportEntireGeometry=off  SerializedFrames=<Frames/>  MarkerShape=Sphere  MarkerSize=10  GraphicType=FromGeometry  InertiaType=CalculateFromGeometry  Mass=1  MassUnits=kg  MomentsOfInertia=[1 1 1]  MomentsOfInertiaUnits=kg*m^2  GraphicOpacity=1.0  ProductsOfInertia=[0 0 0]  ProductsOfInertiaUnits=kg*m^2  GraphicShininess=75  GraphicSpecularColor=[0.5 0.5 0.5 1.0]  GraphicVisPropType=SimpleVisualProperties
  Z axis : sm_lib/Body Elements/Cylindrical Solid  GraphicAmbientColor=[0.15 0.15 0.15 1.0]  BasedOnType=Density  CenterOfMass=[0 0 0]  CenterOfMassUnits=m  GraphicDiffuseColor=[0.0 0.0 1.0]  CylinderLength=0.8  CylinderLengthUnits=m  CylinderRadius=0.01  CylinderRadiusUnits=m  Density=1  DensityUnits=kg/m^3  DoExposeReferenceFrame=on  GraphicEmissiveColor=[0.0 0.0 0.0 1.0]  ExportEntireGeometry=off  SerializedFrames=<Frames/>  MarkerShape=Sphere  MarkerSize=10  GraphicType=FromGeometry  InertiaType=CalculateFromGeometry  Mass=1  MassUnits=kg  MomentsOfInertia=[1 1 1]  MomentsOfInertiaUnits=kg*m^2  GraphicOpacity=1.0  ProductsOfInertia=[0 0 0]  ProductsOfInertiaUnits=kg*m^2  GraphicShininess=75  GraphicSpecularColor=[0.5 0.5 0.5 1.0]  GraphicVisPropType=SimpleVisualProperties
  Rotate_Leg2 : PMIOPort  Side=Left
  connections:
  Z axis/RConn1 -- Rotate_Leg1/RConn1 -- Rotate_Leg2/RConn1 -- Rotate_Leg/RConn1
  Y axis/RConn1 -- Rotate_Leg/LConn1
  Rotate_Leg1/LConn1 -- X Axis/RConn1

== system TripedLibraryMAX/Simplified Model/Parts/Simplified Leg/LCS_gimbal_substitue_joint ==
  LCS_Gimbal_X_Joint : sm_lib/Joints/Revolute Joint  JointMode=Normal  SenseConstraintForce=off  SenseConstraintForceX=off  SenseConstraintForceY=off  SenseConstraintForceZ=off  SenseConstraintTorque=off  SenseConstraintTorqueX=off  SenseConstraintTorqueY=off  SenseConstraintTorqueZ=off  SenseTotalForce=off  SenseTotalForceX=off  SenseTotalForceY=off  SenseTotalForceZ=off  SenseTotalTorque=off  SenseTotalTorqueX=off  SenseTotalTorqueY=off  SenseTotalTorqueZ=off  CompositeWrenchDir=FollowerOnBase  CompositeWrenchFrame=BaseFrame  EquilibriumPosition=0  EquilibriumPositionUnits=deg  SpringStiffness=200  SpringStiffnessUnits=N*m/deg  DampingCoefficient=0  DampingCoefficientUnits=N*m/(deg/s)  TorqueActuationMode=ComputedTorque  MotionActuationMode=InputMotion  SensePosition=on  SenseVelocity=off  SenseAcceleration=off  SenseTorqueForce=off  SenseLowerLimitTorqueForce=off  SenseUpperLimitTorqueForce=off  LowerLimitSpecify=off  LowerLimitBound=-30  LowerLimitBoundUnits=deg  LowerLimitStiffness=1e4  LowerLimitStiffnessUnits=N*m/deg  LowerLimitDamping=10  LowerLimitDampingUnits=N*m/(deg/s)  LowerLimitTransitionRegionWidth=0.1  LowerLimitTransitionRegionWidthUnits=deg  UpperLimitSpecify=off  UpperLimitBound=30  UpperLimitBoundUnits=deg  UpperLimitStiffness=1e4  UpperLimitStiffnessUnits=N*m/deg  UpperLimitDamping=10  UpperLimitDampingUnits=N*m/(deg/s)  UpperLimitTransitionRegionWidth=0.1  UpperLimitTransitionRegionWidthUnits=deg  PositionTargetSpecify=on  PositionTargetPriority=Low  PositionTargetValue=0  PositionTargetValueUnits=deg  VelocityTargetSpecify=off  VelocityTargetPriority=High  VelocityTargetValue=0  VelocityTargetValueUnits=deg/s
  LCS_Gimbal_Y_Joint : sm_lib/Joints/Revolute Joint  JointMode=Normal  SenseConstraintForce=off  SenseConstraintForceX=off  SenseConstraintForceY=off  SenseConstraintForceZ=off  SenseConstraintTorque=off  SenseConstraintTorqueX=off  SenseConstraintTorqueY=off  SenseConstraintTorqueZ=off  SenseTotalForce=off  SenseTotalForceX=off  SenseTotalForceY=off  SenseTotalForceZ=off  SenseTotalTorque=off  SenseTotalTorqueX=off  SenseTotalTorqueY=off  SenseTotalTorqueZ=off  CompositeWrenchDir=FollowerOnBase  CompositeWrenchFrame=BaseFrame  EquilibriumPosition=0  EquilibriumPositionUnits=deg  SpringStiffness=200  SpringStiffnessUnits=N*m/deg  DampingCoefficient=0  DampingCoefficientUnits=N*m/(deg/s)  TorqueActuationMode=ComputedTorque  MotionActuationMode=InputMotion  SensePosition=on  SenseVelocity=off  SenseAcceleration=off  SenseTorqueForce=off  SenseLowerLimitTorqueForce=off  SenseUpperLimitTorqueForce=off  LowerLimitSpecify=off  LowerLimitBound=-30  LowerLimitBoundUnits=deg  LowerLimitStiffness=1e4  LowerLimitStiffnessUnits=N*m/deg  LowerLimitDamping=10  LowerLimitDampingUnits=N*m/(deg/s)  LowerLimitTransitionRegionWidth=0.1  LowerLimitTransitionRegionWidthUnits=deg  UpperLimitSpecify=off  UpperLimitBound=30  UpperLimitBoundUnits=deg  UpperLimitStiffness=1e4  UpperLimitStiffnessUnits=N*m/deg  UpperLimitDamping=10  UpperLimitDampingUnits=N*m/(deg/s)  UpperLimitTransitionRegionWidth=0.1  UpperLimitTransitionRegionWidthUnits=deg  PositionTargetSpecify=on  PositionTargetPriority=Low  PositionTargetValue=0  PositionTargetValueUnits=deg  VelocityTargetSpecify=off  VelocityTargetPriority=High  VelocityTargetValue=0  VelocityTargetValueUnits=deg/s
  LCS_Gimbal_Z_Joint : sm_lib/Joints/Revolute Joint  JointMode=Normal  SenseConstraintForce=off  SenseConstraintForceX=off  SenseConstraintForceY=off  SenseConstraintForceZ=off  SenseConstraintTorque=off  SenseConstraintTorqueX=off  SenseConstraintTorqueY=off  SenseConstraintTorqueZ=off  SenseTotalForce=off  SenseTotalForceX=off  SenseTotalForceY=off  SenseTotalForceZ=off  SenseTotalTorque=off  SenseTotalTorqueX=off  SenseTotalTorqueY=off  SenseTotalTorqueZ=off  CompositeWrenchDir=FollowerOnBase  CompositeWrenchFrame=BaseFrame  EquilibriumPosition=0  EquilibriumPositionUnits=deg  SpringStiffness=200  SpringStiffnessUnits=N*m/deg  DampingCoefficient=0  DampingCoefficientUnits=N*m/(deg/s)  TorqueActuationMode=ComputedTorque  MotionActuationMode=InputMotion  SensePosition=on  SenseVelocity=off  SenseAcceleration=off  SenseTorqueForce=off  SenseLowerLimitTorqueForce=off  SenseUpperLimitTorqueForce=off  LowerLimitSpecify=off  LowerLimitBound=-90  LowerLimitBoundUnits=deg  LowerLimitStiffness=1e4  LowerLimitStiffnessUnits=N*m/deg  LowerLimitDamping=10  LowerLimitDampingUnits=N*m/(deg/s)  LowerLimitTransitionRegionWidth=0.1  LowerLimitTransitionRegionWidthUnits=deg  UpperLimitSpecify=off  UpperLimitBound=90  UpperLimitBoundUnits=deg  UpperLimitStiffness=1e4  UpperLimitStiffnessUnits=N*m/deg  UpperLimitDamping=10  UpperLimitDampingUnits=N*m/(deg/s)  UpperLimitTransitionRegionWidth=0.1  UpperLimitTransitionRegionWidthUnits=deg  PositionTargetSpecify=on  PositionTargetPriority=Low  PositionTargetValue=0  PositionTargetValueUnits=deg  VelocityTargetSpecify=off  VelocityTargetPriority=High  VelocityTargetValue=0  VelocityTargetValueUnits=deg/s
  LCS_X_to_Y : sm_lib/Frames and Transforms/Rigid Transform  TranslationMethod=None  TranslationStandardOffset=0  TranslationLengthUnit=m  TranslationStandardAxis=+Z  TranslationCartesianOffset=[0 0 0]  TranslationROffset=0  TranslationROffsetUnits=m  TranslationZOffset=0  TranslationZOffsetUnits=m  TranslationThetaOffset=0  TranslationThetaOffsetUnits=deg  RotationMethod=StandardAxis  RotationAngleUnits=deg  RotationStandardAxis=+X  RotationAngle=-90.0  RotationArbitraryAxis=[0 0 1]  FollAlignAxisA=+X  BaseAlignAxisA=+Y  FollAlignAxisB=+Y  BaseAlignAxisB=+Z  RotationSequenceAxes=FollowerAxes  RotationSequence=XYX  RotationSequenceAngles=[0 0 0]  RotationSequenceAnglesUnits=deg  RotationMatrix=[1 0 0; 0 1 0; 0 0 1]
  LCS_Y_to_Z : sm_lib/Frames and Transforms/Rigid Transform  TranslationMethod=None  TranslationStandardOffset=0  TranslationLengthUnit=m  TranslationStandardAxis=+Z  TranslationCartesianOffset=[0 0 0]  TranslationROffset=0  TranslationROffsetUnits=m  TranslationZOffset=0  TranslationZOffsetUnits=m  TranslationThetaOffset=0  TranslationThetaOffsetUnits=deg  RotationMethod=RotationSequence  RotationAngleUnits=deg  RotationStandardAxis=+Z  RotationAngle=0.0  RotationArbitraryAxis=[0 0 1]  FollAlignAxisA=+X  BaseAlignAxisA=+Y  FollAlignAxisB=+Y  BaseAlignAxisB=+Z  RotationSequenceAxes=FollowerAxes  RotationSequence=XYX  RotationSequenceAngles=[90 -90 0]  RotationSequenceAnglesUnits=deg  RotationMatrix=[1 0 0; 0 1 0; 0 0 1]
  LCS_Z_to_X : sm_lib/Frames and Transforms/Rigid Transform  TranslationMethod=None  TranslationStandardOffset=0  TranslationLengthUnit=m  TranslationStandardAxis=+Z  TranslationCartesianOffset=[0 0 0]  TranslationROffset=0  TranslationROffsetUnits=m  TranslationZOffset=0  TranslationZOffsetUnits=m  TranslationThetaOffset=0  TranslationThetaOffsetUnits=deg  RotationMethod=StandardAxis  RotationAngleUnits=deg  RotationStandardAxis=+Y  RotationAngle=90.0  RotationArbitraryAxis=[0 0 1]  FollAlignAxisA=+X  BaseAlignAxisA=+Y  FollAlignAxisB=+Y  BaseAlignAxisB=+Z  RotationSequenceAxes=FollowerAxes  RotationSequence=XYX  RotationSequenceAngles=[0 0 0]  RotationSequenceAnglesUnits=deg  RotationMatrix=[1 0 0; 0 1 0; 0 0 1]
  B : PMIOPort  Side=Left
  qx : PMIOPort  Port=2  Side=Left
  qy : PMIOPort  Port=3  Side=Left
  qz : PMIOPort  Port=4  Side=Left
  F : PMIOPort  Port=5  Side=Right
  qx_out : PMIOPort  Port=6  Side=Right
  qy_out : PMIOPort  Port=7  Side=Right
  qz_out : PMIOPort  Port=8  Side=Right
  connections:
  LCS_X_to_Y/LConn1 -- LCS_Gimbal_X_Joint/RConn1
  LCS_Z_to_X/RConn1 -- LCS_Gimbal_X_Joint/LConn1
  LCS_Y_to_Z/RConn1 -- LCS_Gimbal_Z_Joint/LConn1
  LCS_X_to_Y/RConn1 -- LCS_Gimbal_Y_Joint/LConn1
  LCS_Gimbal_Z_Joint/RConn2 -- qz_out/RConn1
  qx/RConn1 -- LCS_Gimbal_X_Joint/LConn2
  LCS_Gimbal_Y_Joint/RConn1 -- LCS_Y_to_Z/LConn1
  LCS_Z_to_X/LConn1 -- B/RConn1
  qz/RConn1 -- LCS_Gimbal_Z_Joint/LConn2
  LCS_Gimbal_X_Joint/RConn2 -- qx_out/RConn1
  F/RConn1 -- LCS_Gimbal_Z_Joint/RConn1
  LCS_Gimbal_Y_Joint/RConn2 -- qy_out/RConn1
  qy/RConn1 -- LCS_Gimbal_Y_Joint/LConn2

== system TripedLibraryMAX/Simplified Model/Simplified TriPed/Chassis/visualize CCS ==
  Rotate_Leg : sm_lib/Frames and Transforms/Rigid Transform  TranslationMethod=None  TranslationStandardOffset=0  TranslationLengthUnit=m  TranslationStandardAxis=+Z  TranslationCartesianOffset=[0 0 0]  TranslationROffset=0  TranslationROffsetUnits=m  TranslationZOffset=0  TranslationZOffsetUnits=m  TranslationThetaOffset=0  TranslationThetaOffsetUnits=deg  RotationMethod=StandardAxis  RotationAngleUnits=deg  RotationStandardAxis=+X  RotationAngle=90  RotationArbitraryAxis=[0 0 1]  FollAlignAxisA=+X  BaseAlignAxisA=+Y  FollAlignAxisB=+Y  BaseAlignAxisB=+Z  RotationSequenceAxes=FollowerAxes  RotationSequence=XYX  RotationSequenceAngles=[0 0 0]  RotationSequenceAnglesUnits=deg  RotationMatrix=[1 0 0; 0 1 0; 0 0 1]
  Rotate_Leg1 : sm_lib/Frames and Transforms/Rigid Transform  TranslationMethod=None  TranslationStandardOffset=0  TranslationLengthUnit=m  TranslationStandardAxis=+Z  TranslationCartesianOffset=[0 0 0]  TranslationROffset=0  TranslationROffsetUnits=m  TranslationZOffset=0  TranslationZOffsetUnits=m  TranslationThetaOffset=0  TranslationThetaOffsetUnits=deg  RotationMethod=StandardAxis  RotationAngleUnits=deg  RotationStandardAxis=+Y  RotationAngle=90  RotationArbitraryAxis=[0 0 1]  FollAlignAxisA=+X  BaseAlignAxisA=+Y  FollAlignAxisB=+Y  BaseAlignAxisB=+Z  RotationSequenceAxes=FollowerAxes  RotationSequence=XYX  RotationSequenceAngles=[0 0 0]  RotationSequenceAnglesUnits=deg  RotationMatrix=[1 0 0; 0 1 0; 0 0 1]
  X Axis : sm_lib/Body Elements/Cylindrical Solid  GraphicAmbientColor=[0.15 0.15 0.15 1.0]  BasedOnType=Density  CenterOfMass=[0 0 0]  CenterOfMassUnits=m  GraphicDiffuseColor=[1.0 0.0 0.0]  CylinderLength=0.8  CylinderLengthUnits=m  CylinderRadius=0.01  CylinderRadiusUnits=m  Density=1  DensityUnits=kg/m^3  DoExposeReferenceFrame=on  GraphicEmissiveColor=[0.0 0.0 0.0 1.0]  ExportEntireGeometry=off  SerializedFrames=<Frames/>  MarkerShape=Sphere  MarkerSize=10  GraphicType=FromGeometry  InertiaType=CalculateFromGeometry  Mass=1  MassUnits=kg  MomentsOfInertia=[1 1 1]  MomentsOfInertiaUnits=kg*m^2  GraphicOpacity=1.0  ProductsOfInertia=[0 0 0]  ProductsOfInertiaUnits=kg*m^2  GraphicShininess=75  GraphicSpecularColor=[0.5 0.5 0.5 1.0]  GraphicVisPropType=SimpleVisualProperties
  Y axis : sm_lib/Body Elements/Cylindrical Solid  GraphicAmbientColor=[0.15 0.15 0.15 1.0]  BasedOnType=Density  CenterOfMass=[0 0 0]  CenterOfMassUnits=m  GraphicDiffuseColor=[0.0 1.0 0.0]  CylinderLength=0.8  CylinderLengthUnits=m  CylinderRadius=0.01  CylinderRadiusUnits=m  Density=1  DensityUnits=kg/m^3  DoExposeReferenceFrame=on  GraphicEmissiveColor=[0.0 0.0 0.0 1.0]  ExportEntireGeometry=off  SerializedFrames=<Frames/>  MarkerShape=Sphere  MarkerSize=10  GraphicType=FromGeometry  InertiaType=CalculateFromGeometry  Mass=1  MassUnits=kg  MomentsOfInertia=[1 1 1]  MomentsOfInertiaUnits=kg*m^2  GraphicOpacity=1.0  ProductsOfInertia=[0 0 0]  ProductsOfInertiaUnits=kg*m^2  GraphicShininess=75  GraphicSpecularColor=[0.5 0.5 0.5 1.0]  GraphicVisPropType=SimpleVisualProperties
  Z axis : sm_lib/Body Elements/Cylindrical Solid  GraphicAmbientColor=[0.15 0.15 0.15 1.0]  BasedOnType=Density  CenterOfMass=[0 0 0]  CenterOfMassUnits=m  GraphicDiffuseColor=[0.0 0.0 1.0]  CylinderLength=0.8  CylinderLengthUnits=m  CylinderRadius=0.01  CylinderRadiusUnits=m  Density=1  DensityUnits=kg/m^3  DoExposeReferenceFrame=on  GraphicEmissiveColor=[0.0 0.0 0.0 1.0]  ExportEntireGeometry=off  SerializedFrames=<Frames/>  MarkerShape=Sphere  MarkerSize=10  GraphicType=FromGeometry  InertiaType=CalculateFromGeometry  Mass=1  MassUnits=kg  MomentsOfInertia=[1 1 1]  MomentsOfInertiaUnits=kg*m^2  GraphicOpacity=1.0  ProductsOfInertia=[0 0 0]  ProductsOfInertiaUnits=kg*m^2  GraphicShininess=75  GraphicSpecularColor=[0.5 0.5 0.5 1.0]  GraphicVisPropType=SimpleVisualProperties
  Rotate_Leg2 : PMIOPort  Side=Left
  connections:
  Z axis/RConn1 -- Rotate_Leg1/RConn1 -- Rotate_Leg2/RConn1 -- Rotate_Leg/RConn1
  Y axis/RConn1 -- Rotate_Leg/LConn1
  Rotate_Leg1/LConn1 -- X Axis/RConn1
